simulink model "l8_ver140"  (Simulink 7.9) (continued)
== system l8_ver140 == (continued)
      mask init: same as l8_ver140/adcsnap0
      mask StyleString: popup(bram|dram),popup(1|2|3|4),popup(200|250|266|300|333),edit,popup(8|16|32|64|128),checkbox,checkbox,checkbox,checkbox
      mask CallbackString: snapshot_callback();||||||||
  s5 : Subsystem  mask{mult_latency=6, add_latency=2, bram_length=10}
      mask prompts: mult_latency "Multiplier Latency"; add_latency "Adder Latency"; bram_length "BRAM Length(2^n)"
  s6 : Subsystem  mask{mult_latency=6, add_latency=2, bram_length=10}
      mask prompts: mult_latency "Multiplier Latency"; add_latency "Adder Latency"; bram_length "BRAM Length(2^n)"
  s67snap : Subsystem  Tag=casper:snapshot  AncestorBlock=casper_library_scopes/snapshot  mask(snapshot){storage=bram, dram_dimm=1, dram_clock=200, nsamples=12, data_width=64, offset=off, circap=off, value=off, use_dsp48=on}
      mask prompts: storage "Storage medium"; dram_dimm "DRAM dimm"; dram_clock "DRAM clock rate"; nsamples "Number of Samples (2^?)"; data_width "Data width"; offset "Start delay support"; circap "Circular capture support"; value "Extra value capture support"; use_dsp48 "Use DSP48s to implement counters"
      mask help:
      | Standard snap block, but with the following optional extras;
      |  1) Optionally delay the fabric trigger by a user-specified number of valids.
      |  2) Capture continuously after a trigger until a stop pulse is received. Data is captured into BRAM continuously, with old data being overwritten until a stop command is received. Then stop capturing when memory is filled. This enables you to get some data before and some data after the stop pulse. Use the 'tr_en_cnt' register to determine where you are in your vector. 'Addr' register's MSb now indicates 'busy capturing'.
      |  3) Optionally capture a value on an input port to a register at the same time as the first data sample.
      |  4) Implement counters using DSP48Es to save resources.
      mask init: same as l8_ver140/adcsnap0
      mask StyleString: popup(bram|dram),popup(1|2|3|4),popup(200|250|266|300|333),edit,popup(8|16|32|64|128),checkbox,checkbox,checkbox,checkbox
      mask CallbackString: snapshot_callback();||||||||
  s7 : Subsystem  mask{mult_latency=6, add_latency=2, bram_length=10}
      mask prompts: mult_latency "Multiplier Latency"; add_latency "Adder Latency"; bram_length "BRAM Length(2^n)"
  sg : Subsystem
  ssg : Subsystem
  status : Subsystem  Tag=xps:sw_reg  AncestorBlock=xps_library/software register  mask{numios=1, io_dir=From Processor, io_delay=0, sample_period=1, name1=reg, bitwidth1=32, arith_type1=Unsigned, bin_pt1=0}
      mask prompts: numios "Num I/Os"; io_dir "I/O direction"; io_delay "I/O delay"; sample_period "Sample period"; name1 "Name"; bitwidth1 "Bitwidth"; arith_type1 "Type"; bin_pt1 "Binary pt"
      mask init:
      | swreg_init(gcb);
      mask StyleString: edit,popup(To Processor|From Processor),edit,edit,edit,edit,popup(Unsigned|Signed  (2's comp)|Boolean),edit
      mask CallbackString:
      | t = timer('StartDelay', 0.1); t.TimerFcn = {@swreg_cb, gcb}; start(t);|a = get_param(gcb, 'MaskEnables');
      | if strcmp(get_param(gcb, 'io_dir'), 'To Processor'),
      | a(4) = {'off'};
      | else
      | a(4) = {'on'};
      | end
      | set_param(gcb, 'MaskEnables', a);
      | |||||a = get_param(gcb, 'MaskEnables');
      | if strcmp(get_param(gcb, 'arith_type1'), 'Boolean'),
      | set_param(gcb, 'bitwidth1', '1');
      | set_param(gcb, 'bin_pt1', '0');
      | a(6) = {'off'};
      | a(8) = {'off'};
      | else
      | a(6) = {'on'};
      | a(8) = {'on'};
      | end
      | set_param(gcb, 'MaskEnables', a);
      | |
      mask TabNameString: Setup,Setup,Setup,Setup,IO1,IO1,IO1,IO1
  subband_sampler : Subsystem  mask{subband=1}
      mask prompts: subband "Sub-band number (1 - 8)"
      mask init:
      | if ((subband < 1) || (subband > 8))
      |     err_msg = sprintf('Sub-band number should be in [1, 8]!');
      |     errordlg(err_msg);
      | end
      | 
      | if (mod(subband, 2) == 1)   % odd
      |     offset = 32 * subband;
      | else                        % even
      |     offset = 32 * (subband - 2);
      | end
  trig : Subsystem  Tag=xps:sw_reg  AncestorBlock=xps_library/software register  mask{numios=1, io_dir=From Processor, io_delay=0, sample_period=1, name1=reg, bitwidth1=32, arith_type1=Unsigned, bin_pt1=0}
      mask prompts: numios "Num I/Os"; io_dir "I/O direction"; io_delay "I/O delay"; sample_period "Sample period"; name1 "Name"; bitwidth1 "Bitwidth"; arith_type1 "Type"; bin_pt1 "Binary pt"
      mask init:
      | swreg_init(gcb);
      mask StyleString: edit,popup(To Processor|From Processor),edit,edit,edit,edit,popup(Unsigned|Signed  (2's comp)|Boolean),edit
      mask CallbackString:
      | t = timer('StartDelay', 0.1); t.TimerFcn = {@swreg_cb, gcb}; start(t);|a = get_param(gcb, 'MaskEnables');
      | if strcmp(get_param(gcb, 'io_dir'), 'To Processor'),
      | a(4) = {'off'};
      | else
      | a(4) = {'on'};
      | end
      | set_param(gcb, 'MaskEnables', a);
      | |||||a = get_param(gcb, 'MaskEnables');
      | if strcmp(get_param(gcb, 'arith_type1'), 'Boolean'),
      | set_param(gcb, 'bitwidth1', '1');
      | set_param(gcb, 'bin_pt1', '0');
      | a(6) = {'off'};
      | a(8) = {'off'};
      | else
      | a(6) = {'on'};
      | a(8) = {'on'};
      | end
      | set_param(gcb, 'MaskEnables', a);
      | |
      mask TabNameString: Setup,Setup,Setup,Setup,IO1,IO1,IO1,IO1
  tvg_en : Subsystem  Tag=xps:sw_reg  AncestorBlock=xps_library/software register  mask{numios=1, io_dir=From Processor, io_delay=0, sample_period=1, name1=reg, bitwidth1=32, arith_type1=Unsigned, bin_pt1=0}
      mask prompts: numios "Num I/Os"; io_dir "I/O direction"; io_delay "I/O delay"; sample_period "Sample period"; name1 "Name"; bitwidth1 "Bitwidth"; arith_type1 "Type"; bin_pt1 "Binary pt"
      mask init:
      | swreg_init(gcb);
      mask StyleString: edit,popup(To Processor|From Processor),edit,edit,edit,edit,popup(Unsigned|Signed  (2's comp)|Boolean),edit
      mask CallbackString:
      | t = timer('StartDelay', 0.1); t.TimerFcn = {@swreg_cb, gcb}; start(t);|a = get_param(gcb, 'MaskEnables');
      | if strcmp(get_param(gcb, 'io_dir'), 'To Processor'),
      | a(4) = {'off'};
      | else
      | a(4) = {'on'};
      | end
      | set_param(gcb, 'MaskEnables', a);
      | |||||a = get_param(gcb, 'MaskEnables');
      | if strcmp(get_param(gcb, 'arith_type1'), 'Boolean'),
      | set_param(gcb, 'bitwidth1', '1');
      | set_param(gcb, 'bin_pt1', '0');
      | a(6) = {'off'};
      | a(8) = {'off'};
      | else
      | a(6) = {'on'};
      | a(8) = {'on'};
      | end
      | set_param(gcb, 'MaskEnables', a);
      | |
      mask TabNameString: Setup,Setup,Setup,Setup,IO1,IO1,IO1,IO1
  valid_gen : Subsystem
  xblocks : Subsystem  AncestorBlock=gbt_library/gen_rcs  mask(gen_rcs){lib_name=xblocks_devel, lib_src=git}
      mask prompts: lib_name "Library name"; lib_src "source of revision info"
      mask help:
      | Embeds revision control information for the user design and libraries.
      | On failure to locate specified revision information, falls back to
      | timestamps (current time for libraries).
      | A user specified value is also stored.
      mask init:
      | gen_rcs_init(gcb, ...
      |     'lib_name', lib_name, ...
      |     'lib_src', lib_src);
      settings: AttributesFormatString=|
      | /export/home/ptcs/scratch/models/gjones/xblocks_devel/xblocks_library
      | 8A521D5 : dirty
      mask StyleString: edit,popup(timestamp|git|svn)
  wires:
  Delay54/1 -> [pol1_a0]
  Delay/1 -> converter/1(u8_0)
  Delay2/1 -> converter1/1(u8_0)
  Delay3/1 -> converter2/1(u8_0)
  Delay4/1 -> converter3/1(u8_0)
  Delay5/1 -> converter4/1(u8_0)
  Delay6/1 -> converter5/1(u8_0)
  Delay7/1 -> converter6/1(u8_0)
  Delay8/1 -> converter7/1(u8_0)
  converter/1(s8_7) -> Delay54/1
  converter1/1(s8_7) -> Delay67/1
  converter2/1(s8_7) -> Delay68/1
  converter3/1(s8_7) -> Delay69/1
  converter4/1(s8_7) -> Delay70/1
  converter5/1(s8_7) -> Delay74/1
  converter6/1(s8_7) -> Delay75/1
  converter7/1(s8_7) -> Delay76/1
  Counter/1 -> Slice2/1
  Slice2/1 -> edge_detect1/1
  edge_detect1/1 -> [cic_sync]
  gbe0_overflow/1(sim_out) -> Terminator5/1
  Constant1/1 -> dest_ip/1(sim_reg)
  Constant7/1 -> dest_port/1(sim_reg)
  dest_ip/1(in_reg) -> [dest_ip]
  dest_port/1(in_reg) -> [dest_port]
  [dest_ip] -> gbe0/4
  [dest_port] -> Slice4/1
  Constant2/1 -> status/1(sim_reg)
  status/1(in_reg) -> [status]
  packetizer/3(pkt_eof) -> gbe0/6, [pkt_eof]
  [overflow] -> gbe0_overflow/1(out_reg)
  [spead_rst] -> packetizer/1(reset)
  [status_bits] -> packetizer/2(status)
  Reinterpret8/1 -> [cic_pol1_re], Slice3/1
  Slice3/1 -> [s1_pol1_re]
  Slice5/1 -> [s1_pol1_im]
  Slice6/1 -> [s1_pol2_re]
  Slice7/1 -> [s1_pol2_im]
  reset/1(in_reg) -> [reset]  "reset"
  Slice4/1 -> gbe0/5
  gbe0/6 -> Terminator4/1
  gbe0/7 -> Terminator6/1
  gbe0/8 -> Terminator7/1
  gbe0/9 -> Terminator8/1
  gbe0/10 -> Terminator11/1
  gbe0/11 -> Terminator3/1
  Constant11/1 -> gbe0/7
  gbe0/1 -> Terminator14/1
  gbe0/2 -> Terminator15/1
  gbe0/3 -> Terminator16/1
  gbe0/4 -> Terminator17/1
  gbe0/5 -> negedge_delay/1
  Constant12/1 -> gbe0/8
  gbe0/12 -> Terminator12/1
  [gbe_rst] -> gbe0/1
  negedge_delay/1 -> [overflow]
  valid_gen/1(valid) -> [valid]
  Constant10/1 -> reset/1(sim_reg)
  Constant31/1 -> Register18/1
  sg/2(pps_out) -> Register18/3, [master_rst1]
  Register18/1 -> Inverter2/1, Inverter1/1, [data_enable]  "data_enable"
  arm/1(in_reg) -> Slice8/1
  Pulse Generator/1 -> arm/1(sim_reg)
  Inverter1/1 -> sg/2(pps_en)
  edge_detect2/1 -> Logical3/2
  Slice11/1 -> Logical3/1
  Slice9/1 -> Logical/2
  Logical3/1 -> Logical/3
  [arm] -> Register18/2
  [adc_sync] -> sg/1(pps)
  sg/1(sync_out) -> [sync_out]
  Inverter2/1 -> [spead_rst]  "spead_rst"
  Logical/1 -> [gbe_rst]  "gbe_rst"
  [overflow] -> edge_detect2/1
  [reset] -> Slice9/1, Slice11/1
  Delay21/1 -> Slice10/1
  Slice10/1 -> edge_detect/1
  edge_detect/1 -> [adc_sync]  "adc_sync"
  [spead_rst] -> valid_gen/2(reset)
  Slice8/1 -> [arm]  "arm"
  [arm] -> edge_detect4/1
  [valid] -> Register268/1
  [data_enable] -> Register267/1
  reshaper/1(data_out) -> packetizer/3(data), [reshaper_dout]
  reshaper/2(data_valid) -> packetizer/4(data_valid), [reshaper_valid]
  Reinterpret9/1 -> Slice5/1, [cic_pol1_im]
  Reinterpret10/1 -> [cic_pol2_re], Slice6/1
  Reinterpret11/1 -> Slice7/1, [cic_pol2_im]
  Constant4/1 -> trig/1(sim_reg)
  trig/1(in_reg) -> Slice19/1
  packetizer/1(pkt_data) -> gbe0/2, [pktizer_dout]
  packetizer/2(pkt_valid) -> [pkt_valid], Logical1/2
  [s0_pol1_re] -> Concat1/1
  [s0_pol1_im] -> Concat1/2
  [s0_pol2_re] -> Concat1/3
  [s0_pol2_im] -> Concat1/4
  Concat6/1 -> Concat/1, s67snap/1(din)
  Concat5/1 -> Concat/2, s45snap/1(din)
  Concat2/1 -> Concat/3, s23snap/1(din)
  Concat1/1 -> Concat/4, s01snap/1(din)
  Delay38/1 -> [pol2_a0]
  Delay40/1 -> [pol2_a2]
  Delay41/1 -> [pol2_a4]
  Delay42/1 -> [pol2_a6]
  Delay44/1 -> [pol2_a8]
  Delay45/1 -> [pol2_a10]
  Delay46/1 -> [pol2_a12]
  Delay47/1 -> [pol2_a14]
  Delay25/1 -> converter8/1(u8_0)
  Delay33/1 -> converter9/1(u8_0)
  Delay35/1 -> converter10/1(u8_0)
  Delay36/1 -> converter11/1(u8_0)
  Delay37/1 -> converter12/1(u8_0)
  Delay39/1 -> converter13/1(u8_0)
  Delay43/1 -> converter14/1(u8_0)
  Delay48/1 -> converter15/1(u8_0)
  converter8/1(s8_7) -> Delay38/1
  converter9/1(s8_7) -> Delay40/1
  converter10/1(s8_7) -> Delay41/1
  converter11/1(s8_7) -> Delay42/1
  converter12/1(s8_7) -> Delay44/1
  converter13/1(s8_7) -> Delay45/1
  converter14/1(s8_7) -> Delay46/1
  converter15/1(s8_7) -> Delay47/1
  asiaa_adc5g/2(a1) -> Delay9/1
  asiaa_adc5g/4(a3) -> Delay13/1
  asiaa_adc5g/6(a5) -> Delay15/1
  asiaa_adc5g/8(a7) -> Delay17/1
  asiaa_adc5g/10(a9) -> Delay26/1
  asiaa_adc5g/12(a11) -> Delay28/1
  asiaa_adc5g/14(a13) -> Delay29/1
  asiaa_adc5g/16(a15) -> Delay62/1
  asiaa_adc5g/1(a0) -> Delay/1
  asiaa_adc5g/15(a14) -> Delay8/1
  asiaa_adc5g/13(a12) -> Delay7/1
  asiaa_adc5g/11(a10) -> Delay6/1
  asiaa_adc5g/7(a6) -> Delay4/1
  asiaa_adc5g/5(a4) -> Delay3/1
  asiaa_adc5g/3(a2) -> Delay2/1
  asiaa_adc5g/9(a8) -> Delay5/1
  asiaa_adc5g/17(sync_out) -> Delay21/1
  asiaa_adc5g1/1(a0) -> Delay25/1
  asiaa_adc5g1/2(a1) -> Delay31/1
  asiaa_adc5g1/3(a2) -> Delay33/1
  asiaa_adc5g1/4(a3) -> Delay34/1
  asiaa_adc5g1/5(a4) -> Delay35/1
  asiaa_adc5g1/6(a5) -> Delay50/1
  asiaa_adc5g1/7(a6) -> Delay36/1
  asiaa_adc5g1/8(a7) -> Delay52/1
  asiaa_adc5g1/9(a8) -> Delay37/1
  asiaa_adc5g1/10(a9) -> Delay55/1
  asiaa_adc5g1/11(a10) -> Delay39/1
  asiaa_adc5g1/12(a11) -> Delay57/1
  asiaa_adc5g1/13(a12) -> Delay43/1
  asiaa_adc5g1/14(a13) -> Delay59/1
  asiaa_adc5g1/15(a14) -> Delay48/1
  asiaa_adc5g1/16(a15) -> Delay64/1
  Delay11/1 -> [pol1_a1]
  Delay9/1 -> converter16/1(u8_0)
  converter16/1(s8_7) -> Delay11/1
  Delay13/1 -> converter17/1(u8_0)
  converter17/1(s8_7) -> Delay12/1
  Delay15/1 -> converter18/1(u8_0)
  converter18/1(s8_7) -> Delay14/1
  Delay17/1 -> converter19/1(u8_0)
  converter19/1(s8_7) -> Delay16/1
  Delay26/1 -> converter20/1(u8_0)
  converter20/1(s8_7) -> Delay23/1
  Delay28/1 -> converter21/1(u8_0)
  converter21/1(s8_7) -> Delay27/1
  Delay29/1 -> converter22/1(u8_0)
  converter22/1(s8_7) -> Delay30/1
  Delay32/1 -> [pol2_a1]
  Delay31/1 -> converter23/1(u8_0)
  converter23/1(s8_7) -> Delay32/1
  Delay49/1 -> [pol2_a3]
  Delay34/1 -> converter24/1(u8_0)
  converter24/1(s8_7) -> Delay49/1
  Delay51/1 -> [pol2_a5]
  Delay50/1 -> converter25/1(u8_0)
  converter25/1(s8_7) -> Delay51/1
  Delay53/1 -> [pol2_a7]
  Delay52/1 -> converter26/1(u8_0)
  converter26/1(s8_7) -> Delay53/1
  Delay56/1 -> [pol2_a9]
  Delay55/1 -> converter27/1(u8_0)
  converter27/1(s8_7) -> Delay56/1
  Delay58/1 -> [pol2_a11]
  Delay57/1 -> converter28/1(u8_0)
  converter28/1(s8_7) -> Delay58/1
  Delay60/1 -> [pol2_a13]
  Delay59/1 -> converter29/1(u8_0)
  converter29/1(s8_7) -> Delay60/1
  Delay62/1 -> converter30/1(u8_0)
  converter30/1(s8_7) -> Delay61/1
  Delay63/1 -> [pol2_a15]
  Delay64/1 -> converter31/1(u8_0)
  converter31/1(s8_7) -> Delay63/1
  s1/2(pol1_im) -> Reinterpret9/1
  s1/1(pol1_re) -> Reinterpret8/1
  s1/3(pol2_re) -> Reinterpret10/1
  s1/4(pol2_im) -> Reinterpret11/1
  [cic_sync] -> Register34/1
  [pol1_a0] -> Register1/1
  Constant23/1 -> adcsnap0/2(we)
  bus_create1/1(bus_out) -> adcsnap0/1(din)
  Constant24/1 -> adcsnap1/2(we)
  bus_create2/1(bus_out) -> adcsnap1/1(din)
  [pkt_valid] -> packetizer_snap/2(we)
  [pktizer_dout] -> packetizer_snap/1(din)
  Slice19/1 -> edge_detect3/1
  edge_detect3/1 -> [trig]
  [trig] -> adcsnap0/3(trig)
  [trig] -> adcsnap1/3(trig)
  [trig] -> packetizer_snap/3(trig)
  [s1_pol1_re] -> Concat1/5
  [s1_pol1_im] -> Concat1/6
  [s1_pol2_re] -> Concat1/7
  [s1_pol2_im] -> Concat1/8
  [s2_pol1_re] -> Concat2/1
  [s2_pol1_im] -> Concat2/2
  [s2_pol2_re] -> Concat2/3
  [s2_pol2_im] -> Concat2/4
  [s3_pol1_re] -> Concat2/5
  [s3_pol1_im] -> Concat2/6
  [s3_pol2_re] -> Concat2/7
  [s3_pol2_im] -> Concat2/8
  [s6_pol1_re] -> Concat6/1
  [s6_pol1_im] -> Concat6/2
  [s6_pol2_re] -> Concat6/3
  [s6_pol2_im] -> Concat6/4
  [s7_pol1_re] -> Concat6/5
  [s7_pol1_im] -> Concat6/6
  [s7_pol2_re] -> Concat6/7
  [s7_pol2_im] -> Concat6/8
  [s4_pol1_re] -> Concat5/1
  [s4_pol1_im] -> Concat5/2
  [s4_pol2_re] -> Concat5/3
  [s4_pol2_im] -> Concat5/4
  [s5_pol1_re] -> Concat5/5
  [s5_pol1_im] -> Concat5/6
  [s5_pol2_re] -> Concat5/7
  [s5_pol2_im] -> Concat5/8
  Constant3/1 -> mode_sel/1(sim_reg)
  mode_sel/1(in_reg) -> Slice40/1
  Slice40/1 -> [mode_sel]
  [mode_sel] -> valid_gen/1(mode_sel)
  Mux/1 -> Register266/1
  [mode_sel] -> Mux/1
  subband_sampler/1(data_out) -> Mux/3
  [mode_sel] -> packetizer/5(mode_sel)
  Delay67/1 -> [pol1_a2]
  Delay12/1 -> [pol1_a3]
  Delay68/1 -> [pol1_a4]
  Delay14/1 -> [pol1_a5]
  Delay69/1 -> [pol1_a6]
  Delay16/1 -> [pol1_a7]
  Delay70/1 -> [pol1_a8]
  Delay23/1 -> [pol1_a9]
  Delay74/1 -> [pol1_a10]
  Delay27/1 -> [pol1_a11]
  Delay75/1 -> [pol1_a12]
  Delay30/1 -> [pol1_a13]
  Delay76/1 -> [pol1_a14]
  Delay61/1 -> [pol1_a15]
  [pol1_a1] -> Register2/1
  [pol1_a2] -> Register3/1
  [pol1_a3] -> Register4/1
  [pol1_a4] -> Register5/1
  [pol1_a5] -> Register6/1
  [pol1_a6] -> Register7/1
  [pol1_a7] -> Register8/1
  [pol1_a8] -> Register9/1
  [pol1_a9] -> Register10/1
  [pol1_a10] -> Register11/1
  [pol1_a11] -> Register12/1
  [pol1_a12] -> Register13/1
  [pol1_a13] -> Register14/1
  [pol1_a14] -> Register15/1
  [pol1_a15] -> Register16/1
  [pol2_a0] -> Register17/1
  [pol2_a1] -> Register26/1
  [pol2_a2] -> Register27/1
  [pol2_a3] -> Register28/1
  [pol2_a4] -> Register29/1
  [pol2_a5] -> Register30/1
  [pol2_a6] -> Register31/1
  [pol2_a7] -> Register32/1
  [pol2_a8] -> Register33/1
  [pol2_a9] -> Register19/1
  [pol2_a10] -> Register20/1
  [pol2_a11] -> Register21/1
  [pol2_a12] -> Register22/1
  [pol2_a13] -> Register23/1
  [pol2_a14] -> Register24/1
  [pol2_a15] -> Register25/1
  [pol1_a0] -> bus_create1/1(in1)
  [pol1_a1] -> bus_create1/2(in2)
  [pol1_a2] -> bus_create1/3(in3)
  [pol1_a3] -> bus_create1/4(in4)
  [pol1_a4] -> bus_create1/5(in5)
  [pol1_a5] -> bus_create1/6(in6)
  [pol1_a6] -> bus_create1/7(in7)
  [pol1_a7] -> bus_create1/8(in8)
  [pol1_a8] -> bus_create1/9(in9)
  [pol1_a9] -> bus_create1/10(in10)
  [pol1_a10] -> bus_create1/11(in11)
  [pol1_a11] -> bus_create1/12(in12)
  [pol1_a12] -> bus_create1/13(in13)
  [pol1_a13] -> bus_create1/14(in14)
  [pol1_a14] -> bus_create1/15(in15)
  [pol1_a15] -> bus_create1/16(in16)
  [pol2_a0] -> bus_create2/1(in1)
  [pol2_a1] -> bus_create2/2(in2)
  [pol2_a2] -> bus_create2/3(in3)
  [pol2_a3] -> bus_create2/4(in4)
  [pol2_a4] -> bus_create2/5(in5)
  [pol2_a5] -> bus_create2/6(in6)
  [pol2_a6] -> bus_create2/7(in7)
  [pol2_a7] -> bus_create2/8(in8)
  [pol2_a8] -> bus_create2/9(in9)
  [pol2_a9] -> bus_create2/10(in10)
  [pol2_a10] -> bus_create2/11(in11)
  [pol2_a11] -> bus_create2/12(in12)
  [pol2_a12] -> bus_create2/13(in13)
  [pol2_a13] -> bus_create2/14(in14)
  [pol2_a14] -> bus_create2/15(in15)
  [pol2_a15] -> bus_create2/16(in16)
  ssg/1(statusbits) -> [status_bits]
  [master_rst1] -> Register/1
  Register/1 -> ssg/1(reset)
  [spec_tick] -> ssg/2(spec_tick)
  [master_rst1] -> Counter1/1
  Counter1/1 -> Slice41/1
  Slice41/1 -> edge_detect5/1(in)
  edge_detect5/1(out) -> [spec_tick]
  Reinterpret3/1 -> Slice1/1
  Slice1/1 -> [s0_pol1_re]
  Slice12/1 -> [s0_pol1_im]
  Slice13/1 -> [s0_pol2_re]
  Slice14/1 -> [s0_pol2_im]
  Reinterpret4/1 -> Slice12/1
  Reinterpret1/1 -> Slice13/1
  Reinterpret2/1 -> Slice14/1
  s0/2(pol1_im) -> Reinterpret4/1
  s0/1(pol1_re) -> Reinterpret3/1
  s0/3(pol2_re) -> Reinterpret1/1
  s0/4(pol2_im) -> Reinterpret2/1
  Reinterpret7/1 -> Slice15/1
  Slice15/1 -> [s2_pol1_re]
  Slice16/1 -> [s2_pol1_im]
  Slice17/1 -> [s2_pol2_re]
  Slice18/1 -> [s2_pol2_im]
  Reinterpret12/1 -> Slice16/1
  Reinterpret5/1 -> Slice17/1
  Reinterpret6/1 -> Slice18/1
  s2/2(pol1_im) -> Reinterpret12/1
  s2/1(pol1_re) -> Reinterpret7/1
  s2/3(pol2_re) -> Reinterpret5/1
  s2/4(pol2_im) -> Reinterpret6/1
  Reinterpret16/1 -> Slice20/1
  Slice20/1 -> [s3_pol1_re]
  Slice21/1 -> [s3_pol1_im]
  Slice22/1 -> [s3_pol2_re]
  Slice23/1 -> [s3_pol2_im]
  Reinterpret13/1 -> Slice21/1
  Reinterpret14/1 -> Slice22/1
  Reinterpret15/1 -> Slice23/1
  s3/2(pol1_im) -> Reinterpret13/1
  s3/1(pol1_re) -> Reinterpret16/1
  s3/3(pol2_re) -> Reinterpret14/1
  s3/4(pol2_im) -> Reinterpret15/1
  Reinterpret20/1 -> Slice24/1
  Slice24/1 -> [s4_pol1_re]
  Slice25/1 -> [s4_pol1_im]
  Slice26/1 -> [s4_pol2_re]
  Slice27/1 -> [s4_pol2_im]
  Reinterpret17/1 -> Slice25/1
  Reinterpret18/1 -> Slice26/1
  Reinterpret19/1 -> Slice27/1
  s4/2(pol1_im) -> Reinterpret17/1
  s4/1(pol1_re) -> Reinterpret20/1
  s4/3(pol2_re) -> Reinterpret18/1
  s4/4(pol2_im) -> Reinterpret19/1
  Reinterpret24/1 -> Slice28/1
  Slice28/1 -> [s5_pol1_re]
  Slice29/1 -> [s5_pol1_im]
  Slice30/1 -> [s5_pol2_re]
  Slice31/1 -> [s5_pol2_im]
  Reinterpret21/1 -> Slice29/1
  Reinterpret22/1 -> Slice30/1
  Reinterpret23/1 -> Slice31/1
  s5/2(pol1_im) -> Reinterpret21/1
  s5/1(pol1_re) -> Reinterpret24/1
  s5/3(pol2_re) -> Reinterpret22/1
  s5/4(pol2_im) -> Reinterpret23/1
  Reinterpret28/1 -> Slice32/1
  Slice32/1 -> [s6_pol1_re]
  Slice33/1 -> [s6_pol1_im]
  Slice34/1 -> [s6_pol2_re]
  Slice35/1 -> [s6_pol2_im]
  Reinterpret25/1 -> Slice33/1
  Reinterpret26/1 -> Slice34/1
  Reinterpret27/1 -> Slice35/1
  s6/2(pol1_im) -> Reinterpret25/1
  s6/1(pol1_re) -> Reinterpret28/1
  s6/3(pol2_re) -> Reinterpret26/1
  s6/4(pol2_im) -> Reinterpret27/1
  Reinterpret32/1 -> Slice36/1
  Slice36/1 -> [s7_pol1_re]
  Slice37/1 -> [s7_pol1_im]
  Slice38/1 -> [s7_pol2_re]
  Slice39/1 -> [s7_pol2_im]
  Reinterpret29/1 -> Slice37/1
  Reinterpret30/1 -> Slice38/1
  Reinterpret31/1 -> Slice39/1
  s7/2(pol1_im) -> Reinterpret29/1
  s7/1(pol1_re) -> Reinterpret32/1
  s7/3(pol2_re) -> Reinterpret30/1
  s7/4(pol2_im) -> Reinterpret31/1
  Register1/1 -> s1/1(pol1_in0)
  Register2/1 -> s1/2(pol1_in1)
  Register3/1 -> s1/3(pol1_in2)
  Register4/1 -> s1/4(pol1_in3)
  Register5/1 -> s1/5(pol1_in4)
  Register6/1 -> s1/6(pol1_in5)
  Register7/1 -> s1/7(pol1_in6)
  Register8/1 -> s1/8(pol1_in7)
  Register9/1 -> s1/9(pol1_in8)
  Register10/1 -> s1/10(pol1_in9)
  Register11/1 -> s1/11(pol1_in10)
  Register12/1 -> s1/12(pol1_in11)
  Register13/1 -> s1/13(pol1_in12)
  Register14/1 -> s1/14(pol1_in13)
  Register15/1 -> s1/15(pol1_in14)
  Register16/1 -> s1/16(pol1_in15)
  Register17/1 -> s1/17(pol2_in0)
  Register26/1 -> s1/18(pol2_in1)
  Register27/1 -> s1/19(pol2_in2)
  Register28/1 -> s1/20(pol2_in3)
  Register29/1 -> s1/21(pol2_in4)
  Register30/1 -> s1/22(pol2_in5)
  Register31/1 -> s1/23(pol2_in6)
  Register32/1 -> s1/24(pol2_in7)
  Register33/1 -> s1/25(pol2_in8)
  Register19/1 -> s1/26(pol2_in9)
  Register20/1 -> s1/27(pol2_in10)
  Register21/1 -> s1/28(pol2_in11)
  Register22/1 -> s1/29(pol2_in12)
  Register23/1 -> s1/30(pol2_in13)
  Register24/1 -> s1/31(pol2_in14)
  Register25/1 -> s1/32(pol2_in15)
  Register34/1 -> s1/33(adc_CIC_sync)
  edge_detect4/1 -> Delay329/1
  Delay329/1 -> Logical/1
  Logical1/1 -> gbe0/3
  [data_enable] -> Logical1/1
  [valid] -> s01snap/2(we)
  [cic_sync] -> Register61/1
  [pol1_a0] -> Register35/1
  [pol1_a1] -> Register45/1
  [pol1_a2] -> Register56/1
  [pol1_a3] -> Register62/1
  [pol1_a4] -> Register63/1
  [pol1_a5] -> Register64/1
  [pol1_a6] -> Register65/1
  [pol1_a7] -> Register66/1
  [pol1_a8] -> Register67/1
  [pol1_a9] -> Register36/1
  [pol1_a10] -> Register37/1
  [pol1_a11] -> Register38/1
  [pol1_a12] -> Register39/1
  [pol1_a13] -> Register40/1
  [pol1_a14] -> Register41/1
  [pol1_a15] -> Register42/1
  [pol2_a0] -> Register43/1
  [pol2_a1] -> Register52/1
  [pol2_a2] -> Register53/1
  [pol2_a3] -> Register54/1
  [pol2_a4] -> Register55/1
  [pol2_a5] -> Register57/1
  [pol2_a6] -> Register58/1
  [pol2_a7] -> Register59/1
  [pol2_a8] -> Register60/1
  [pol2_a9] -> Register44/1
  [pol2_a10] -> Register46/1
  [pol2_a11] -> Register47/1
  [pol2_a12] -> Register48/1
  [pol2_a13] -> Register49/1
  [pol2_a14] -> Register50/1
  [pol2_a15] -> Register51/1
  Register35/1 -> s0/1(pol1_in0)
  Register45/1 -> s0/2(pol1_in1)
  Register56/1 -> s0/3(pol1_in2)
  Register62/1 -> s0/4(pol1_in3)
  Register63/1 -> s0/5(pol1_in4)
  Register64/1 -> s0/6(pol1_in5)
  Register65/1 -> s0/7(pol1_in6)
  Register66/1 -> s0/8(pol1_in7)
  Register67/1 -> s0/9(pol1_in8)
  Register36/1 -> s0/10(pol1_in9)
  Register37/1 -> s0/11(pol1_in10)
  Register38/1 -> s0/12(pol1_in11)
  Register39/1 -> s0/13(pol1_in12)
  Register40/1 -> s0/14(pol1_in13)
  Register41/1 -> s0/15(pol1_in14)
  Register42/1 -> s0/16(pol1_in15)
  Register43/1 -> s0/17(pol2_in0)
  Register52/1 -> s0/18(pol2_in1)
  Register53/1 -> s0/19(pol2_in2)
  Register54/1 -> s0/20(pol2_in3)
  Register55/1 -> s0/21(pol2_in4)
  Register57/1 -> s0/22(pol2_in5)
  Register58/1 -> s0/23(pol2_in6)
  Register59/1 -> s0/24(pol2_in7)
  Register60/1 -> s0/25(pol2_in8)
  Register44/1 -> s0/26(pol2_in9)
  Register46/1 -> s0/27(pol2_in10)
  Register47/1 -> s0/28(pol2_in11)
  Register48/1 -> s0/29(pol2_in12)
  Register49/1 -> s0/30(pol2_in13)
  Register50/1 -> s0/31(pol2_in14)
  Register51/1 -> s0/32(pol2_in15)
  Register61/1 -> s0/33(adc_CIC_sync)
  [cic_sync] -> Register94/1
  [pol1_a0] -> Register68/1
  [pol1_a1] -> Register78/1
  [pol1_a2] -> Register89/1
  [pol1_a3] -> Register95/1
  [pol1_a4] -> Register96/1
  [pol1_a5] -> Register97/1
  [pol1_a6] -> Register98/1
  [pol1_a7] -> Register99/1
  [pol1_a8] -> Register100/1
  [pol1_a9] -> Register69/1
  [pol1_a10] -> Register70/1
  [pol1_a11] -> Register71/1
  [pol1_a12] -> Register72/1
  [pol1_a13] -> Register73/1
  [pol1_a14] -> Register74/1
  [pol1_a15] -> Register75/1
  [pol2_a0] -> Register76/1
  [pol2_a1] -> Register85/1
  [pol2_a2] -> Register86/1
  [pol2_a3] -> Register87/1
  [pol2_a4] -> Register88/1
  [pol2_a5] -> Register90/1
  [pol2_a6] -> Register91/1
  [pol2_a7] -> Register92/1
  [pol2_a8] -> Register93/1
  [pol2_a9] -> Register77/1
  [pol2_a10] -> Register79/1
  [pol2_a11] -> Register80/1
  [pol2_a12] -> Register81/1
  [pol2_a13] -> Register82/1
  [pol2_a14] -> Register83/1
  [pol2_a15] -> Register84/1
  Register68/1 -> s3/1(pol1_in0)
  Register78/1 -> s3/2(pol1_in1)
  Register89/1 -> s3/3(pol1_in2)
  Register95/1 -> s3/4(pol1_in3)
  Register96/1 -> s3/5(pol1_in4)
  Register97/1 -> s3/6(pol1_in5)
  Register98/1 -> s3/7(pol1_in6)
  Register99/1 -> s3/8(pol1_in7)
  Register100/1 -> s3/9(pol1_in8)
  Register69/1 -> s3/10(pol1_in9)
  Register70/1 -> s3/11(pol1_in10)
  Register71/1 -> s3/12(pol1_in11)
  Register72/1 -> s3/13(pol1_in12)
  Register73/1 -> s3/14(pol1_in13)
  Register74/1 -> s3/15(pol1_in14)
  Register75/1 -> s3/16(pol1_in15)
  Register76/1 -> s3/17(pol2_in0)
  Register85/1 -> s3/18(pol2_in1)
  Register86/1 -> s3/19(pol2_in2)
  Register87/1 -> s3/20(pol2_in3)
  Register88/1 -> s3/21(pol2_in4)
  Register90/1 -> s3/22(pol2_in5)
  Register91/1 -> s3/23(pol2_in6)
  Register92/1 -> s3/24(pol2_in7)
  Register93/1 -> s3/25(pol2_in8)
  Register77/1 -> s3/26(pol2_in9)
  Register79/1 -> s3/27(pol2_in10)
  Register80/1 -> s3/28(pol2_in11)
  Register81/1 -> s3/29(pol2_in12)
  Register82/1 -> s3/30(pol2_in13)
  Register83/1 -> s3/31(pol2_in14)
  Register84/1 -> s3/32(pol2_in15)
  Register94/1 -> s3/33(adc_CIC_sync)
  [cic_sync] -> Register128/1
  [pol1_a0] -> Register102/1
  [pol1_a1] -> Register112/1
  [pol1_a2] -> Register123/1
  [pol1_a3] -> Register129/1
  [pol1_a4] -> Register130/1
  [pol1_a5] -> Register131/1
  [pol1_a6] -> Register132/1
  [pol1_a7] -> Register133/1
  [pol1_a8] -> Register101/1
  [pol1_a9] -> Register103/1
  [pol1_a10] -> Register104/1
  [pol1_a11] -> Register105/1
  [pol1_a12] -> Register106/1
  [pol1_a13] -> Register107/1
  [pol1_a14] -> Register108/1
  [pol1_a15] -> Register109/1
  [pol2_a0] -> Register110/1
  [pol2_a1] -> Register119/1
  [pol2_a2] -> Register120/1
  [pol2_a3] -> Register121/1
  [pol2_a4] -> Register122/1
  [pol2_a5] -> Register124/1
  [pol2_a6] -> Register125/1
  [pol2_a7] -> Register126/1
  [pol2_a8] -> Register127/1
  [pol2_a9] -> Register111/1
  [pol2_a10] -> Register113/1
  [pol2_a11] -> Register114/1
  [pol2_a12] -> Register115/1
  [pol2_a13] -> Register116/1
  [pol2_a14] -> Register117/1
  [pol2_a15] -> Register118/1
  Register102/1 -> s2/1(pol1_in0)
  Register112/1 -> s2/2(pol1_in1)
  Register123/1 -> s2/3(pol1_in2)
  Register129/1 -> s2/4(pol1_in3)
  Register130/1 -> s2/5(pol1_in4)
  Register131/1 -> s2/6(pol1_in5)
  Register132/1 -> s2/7(pol1_in6)
  Register133/1 -> s2/8(pol1_in7)
  Register101/1 -> s2/9(pol1_in8)
  Register103/1 -> s2/10(pol1_in9)
  Register104/1 -> s2/11(pol1_in10)
  Register105/1 -> s2/12(pol1_in11)
  Register106/1 -> s2/13(pol1_in12)
  Register107/1 -> s2/14(pol1_in13)
  Register108/1 -> s2/15(pol1_in14)
  Register109/1 -> s2/16(pol1_in15)
  Register110/1 -> s2/17(pol2_in0)
  Register119/1 -> s2/18(pol2_in1)
  Register120/1 -> s2/19(pol2_in2)
  Register121/1 -> s2/20(pol2_in3)
  Register122/1 -> s2/21(pol2_in4)
  Register124/1 -> s2/22(pol2_in5)
  Register125/1 -> s2/23(pol2_in6)
  Register126/1 -> s2/24(pol2_in7)
  Register127/1 -> s2/25(pol2_in8)
  Register111/1 -> s2/26(pol2_in9)
  Register113/1 -> s2/27(pol2_in10)
  Register114/1 -> s2/28(pol2_in11)
  Register115/1 -> s2/29(pol2_in12)
  Register116/1 -> s2/30(pol2_in13)
  Register117/1 -> s2/31(pol2_in14)
  Register118/1 -> s2/32(pol2_in15)
  Register128/1 -> s2/33(adc_CIC_sync)
  [cic_sync] -> Register161/1
  [pol1_a0] -> Register135/1
  [pol1_a1] -> Register145/1
  [pol1_a2] -> Register156/1
  [pol1_a3] -> Register162/1
  [pol1_a4] -> Register163/1
  [pol1_a5] -> Register164/1
  [pol1_a6] -> Register165/1
  [pol1_a7] -> Register166/1
  [pol1_a8] -> Register134/1
  [pol1_a9] -> Register136/1
  [pol1_a10] -> Register137/1
  [pol1_a11] -> Register138/1
  [pol1_a12] -> Register139/1
  [pol1_a13] -> Register140/1
  [pol1_a14] -> Register141/1
  [pol1_a15] -> Register142/1
  [pol2_a0] -> Register143/1
  [pol2_a1] -> Register152/1
  [pol2_a2] -> Register153/1
  [pol2_a3] -> Register154/1
  [pol2_a4] -> Register155/1
  [pol2_a5] -> Register157/1
  [pol2_a6] -> Register158/1
  [pol2_a7] -> Register159/1
  [pol2_a8] -> Register160/1
  [pol2_a9] -> Register144/1
  [pol2_a10] -> Register146/1
  [pol2_a11] -> Register147/1
  [pol2_a12] -> Register148/1
  [pol2_a13] -> Register149/1
  [pol2_a14] -> Register150/1
  [pol2_a15] -> Register151/1
  Register135/1 -> s4/1(pol1_in0)
  Register145/1 -> s4/2(pol1_in1)
  Register156/1 -> s4/3(pol1_in2)
  Register162/1 -> s4/4(pol1_in3)
  Register163/1 -> s4/5(pol1_in4)
  Register164/1 -> s4/6(pol1_in5)
  Register165/1 -> s4/7(pol1_in6)
  Register166/1 -> s4/8(pol1_in7)
  Register134/1 -> s4/9(pol1_in8)
  Register136/1 -> s4/10(pol1_in9)
  Register137/1 -> s4/11(pol1_in10)
  Register138/1 -> s4/12(pol1_in11)
  Register139/1 -> s4/13(pol1_in12)
  Register140/1 -> s4/14(pol1_in13)
  Register141/1 -> s4/15(pol1_in14)
  Register142/1 -> s4/16(pol1_in15)
  Register143/1 -> s4/17(pol2_in0)
  Register152/1 -> s4/18(pol2_in1)
  Register153/1 -> s4/19(pol2_in2)
  Register154/1 -> s4/20(pol2_in3)
  Register155/1 -> s4/21(pol2_in4)
  Register157/1 -> s4/22(pol2_in5)
  Register158/1 -> s4/23(pol2_in6)
  Register159/1 -> s4/24(pol2_in7)
  Register160/1 -> s4/25(pol2_in8)
  Register144/1 -> s4/26(pol2_in9)
  Register146/1 -> s4/27(pol2_in10)
  Register147/1 -> s4/28(pol2_in11)
  Register148/1 -> s4/29(pol2_in12)
  Register149/1 -> s4/30(pol2_in13)
  Register150/1 -> s4/31(pol2_in14)
  Register151/1 -> s4/32(pol2_in15)
  Register161/1 -> s4/33(adc_CIC_sync)
  [cic_sync] -> Register194/1
  [pol1_a0] -> Register168/1
  [pol1_a1] -> Register178/1
  [pol1_a2] -> Register189/1
  [pol1_a3] -> Register195/1
  [pol1_a4] -> Register196/1
  [pol1_a5] -> Register197/1
  [pol1_a6] -> Register198/1
  [pol1_a7] -> Register199/1
  [pol1_a8] -> Register167/1
  [pol1_a9] -> Register169/1
  [pol1_a10] -> Register170/1
  [pol1_a11] -> Register171/1
  [pol1_a12] -> Register172/1
  [pol1_a13] -> Register173/1
  [pol1_a14] -> Register174/1
  [pol1_a15] -> Register175/1
  [pol2_a0] -> Register176/1
  [pol2_a1] -> Register185/1
  [pol2_a2] -> Register186/1
  [pol2_a3] -> Register187/1
  [pol2_a4] -> Register188/1
  [pol2_a5] -> Register190/1
  [pol2_a6] -> Register191/1
  [pol2_a7] -> Register192/1
  [pol2_a8] -> Register193/1
  [pol2_a9] -> Register177/1
  [pol2_a10] -> Register179/1
  [pol2_a11] -> Register180/1
  [pol2_a12] -> Register181/1
  [pol2_a13] -> Register182/1
  [pol2_a14] -> Register183/1
  [pol2_a15] -> Register184/1
  Register168/1 -> s5/1(pol1_in0)
  Register178/1 -> s5/2(pol1_in1)
  Register189/1 -> s5/3(pol1_in2)
  Register195/1 -> s5/4(pol1_in3)
  Register196/1 -> s5/5(pol1_in4)
  Register197/1 -> s5/6(pol1_in5)
  Register198/1 -> s5/7(pol1_in6)
  Register199/1 -> s5/8(pol1_in7)
  Register167/1 -> s5/9(pol1_in8)
  Register169/1 -> s5/10(pol1_in9)
  Register170/1 -> s5/11(pol1_in10)
  Register171/1 -> s5/12(pol1_in11)
  Register172/1 -> s5/13(pol1_in12)
  Register173/1 -> s5/14(pol1_in13)
  Register174/1 -> s5/15(pol1_in14)
  Register175/1 -> s5/16(pol1_in15)
  Register176/1 -> s5/17(pol2_in0)
  Register185/1 -> s5/18(pol2_in1)
  Register186/1 -> s5/19(pol2_in2)
  Register187/1 -> s5/20(pol2_in3)
  Register188/1 -> s5/21(pol2_in4)
  Register190/1 -> s5/22(pol2_in5)
  Register191/1 -> s5/23(pol2_in6)
  Register192/1 -> s5/24(pol2_in7)
  Register193/1 -> s5/25(pol2_in8)
  Register177/1 -> s5/26(pol2_in9)
  Register179/1 -> s5/27(pol2_in10)
  Register180/1 -> s5/28(pol2_in11)
  Register181/1 -> s5/29(pol2_in12)
  Register182/1 -> s5/30(pol2_in13)
  Register183/1 -> s5/31(pol2_in14)
  Register184/1 -> s5/32(pol2_in15)
  Register194/1 -> s5/33(adc_CIC_sync)
  [cic_sync] -> Register227/1
  [pol1_a0] -> Register201/1
  [pol1_a1] -> Register211/1
  [pol1_a2] -> Register222/1
  [pol1_a3] -> Register228/1
  [pol1_a4] -> Register229/1
  [pol1_a5] -> Register230/1
  [pol1_a6] -> Register231/1
  [pol1_a7] -> Register232/1
  [pol1_a8] -> Register200/1
  [pol1_a9] -> Register202/1
  [pol1_a10] -> Register203/1
  [pol1_a11] -> Register204/1
  [pol1_a12] -> Register205/1
  [pol1_a13] -> Register206/1
  [pol1_a14] -> Register207/1
  [pol1_a15] -> Register208/1
  [pol2_a0] -> Register209/1
  [pol2_a1] -> Register218/1
  [pol2_a2] -> Register219/1
  [pol2_a3] -> Register220/1
  [pol2_a4] -> Register221/1
  [pol2_a5] -> Register223/1
  [pol2_a6] -> Register224/1
  [pol2_a7] -> Register225/1
  [pol2_a8] -> Register226/1
  [pol2_a9] -> Register210/1
  [pol2_a10] -> Register212/1
  [pol2_a11] -> Register213/1
  [pol2_a12] -> Register214/1
  [pol2_a13] -> Register215/1
  [pol2_a14] -> Register216/1
  [pol2_a15] -> Register217/1
  Register201/1 -> s6/1(pol1_in0)
  Register211/1 -> s6/2(pol1_in1)
  Register222/1 -> s6/3(pol1_in2)
  Register228/1 -> s6/4(pol1_in3)
  Register229/1 -> s6/5(pol1_in4)
  Register230/1 -> s6/6(pol1_in5)
  Register231/1 -> s6/7(pol1_in6)
  Register232/1 -> s6/8(pol1_in7)
  Register200/1 -> s6/9(pol1_in8)
  Register202/1 -> s6/10(pol1_in9)
  Register203/1 -> s6/11(pol1_in10)
  Register204/1 -> s6/12(pol1_in11)
  Register205/1 -> s6/13(pol1_in12)
  Register206/1 -> s6/14(pol1_in13)
  Register207/1 -> s6/15(pol1_in14)
  Register208/1 -> s6/16(pol1_in15)
  Register209/1 -> s6/17(pol2_in0)
  Register218/1 -> s6/18(pol2_in1)
  Register219/1 -> s6/19(pol2_in2)
  Register220/1 -> s6/20(pol2_in3)
  Register221/1 -> s6/21(pol2_in4)
  Register223/1 -> s6/22(pol2_in5)
  Register224/1 -> s6/23(pol2_in6)
  Register225/1 -> s6/24(pol2_in7)
  Register226/1 -> s6/25(pol2_in8)
  Register210/1 -> s6/26(pol2_in9)
  Register212/1 -> s6/27(pol2_in10)
  Register213/1 -> s6/28(pol2_in11)
  Register214/1 -> s6/29(pol2_in12)
  Register215/1 -> s6/30(pol2_in13)
  Register216/1 -> s6/31(pol2_in14)
  Register217/1 -> s6/32(pol2_in15)
  Register227/1 -> s6/33(adc_CIC_sync)
  [cic_sync] -> Register260/1
  [pol1_a0] -> Register234/1
  [pol1_a1] -> Register244/1
  [pol1_a2] -> Register255/1
  [pol1_a3] -> Register261/1
  [pol1_a4] -> Register262/1
  [pol1_a5] -> Register263/1
  [pol1_a6] -> Register264/1
  [pol1_a7] -> Register265/1
  [pol1_a8] -> Register233/1
  [pol1_a9] -> Register235/1
  [pol1_a10] -> Register236/1
  [pol1_a11] -> Register237/1
  [pol1_a12] -> Register238/1
  [pol1_a13] -> Register239/1
  [pol1_a14] -> Register240/1
  [pol1_a15] -> Register241/1
  [pol2_a0] -> Register242/1
  [pol2_a1] -> Register251/1
  [pol2_a2] -> Register252/1
  [pol2_a3] -> Register253/1
  [pol2_a4] -> Register254/1
  [pol2_a5] -> Register256/1
  [pol2_a6] -> Register257/1
  [pol2_a7] -> Register258/1
  [pol2_a8] -> Register259/1
  [pol2_a9] -> Register243/1
  [pol2_a10] -> Register245/1
  [pol2_a11] -> Register246/1
  [pol2_a12] -> Register247/1
  [pol2_a13] -> Register248/1
  [pol2_a14] -> Register249/1
  [pol2_a15] -> Register250/1
  Register234/1 -> s7/1(pol1_in0)
  Register244/1 -> s7/2(pol1_in1)
  Register255/1 -> s7/3(pol1_in2)
  Register261/1 -> s7/4(pol1_in3)
  Register262/1 -> s7/5(pol1_in4)
  Register263/1 -> s7/6(pol1_in5)
  Register264/1 -> s7/7(pol1_in6)
  Register265/1 -> s7/8(pol1_in7)
  Register233/1 -> s7/9(pol1_in8)
  Register235/1 -> s7/10(pol1_in9)
  Register236/1 -> s7/11(pol1_in10)
  Register237/1 -> s7/12(pol1_in11)
  Register238/1 -> s7/13(pol1_in12)
  Register239/1 -> s7/14(pol1_in13)
  Register240/1 -> s7/15(pol1_in14)
  Register241/1 -> s7/16(pol1_in15)
  Register242/1 -> s7/17(pol2_in0)
  Register251/1 -> s7/18(pol2_in1)
  Register252/1 -> s7/19(pol2_in2)
  Register253/1 -> s7/20(pol2_in3)
  Register254/1 -> s7/21(pol2_in4)
  Register256/1 -> s7/22(pol2_in5)
  Register257/1 -> s7/23(pol2_in6)
  Register258/1 -> s7/24(pol2_in7)
  Register259/1 -> s7/25(pol2_in8)
  Register243/1 -> s7/26(pol2_in9)
  Register245/1 -> s7/27(pol2_in10)
  Register246/1 -> s7/28(pol2_in11)
  Register247/1 -> s7/29(pol2_in12)
  Register248/1 -> s7/30(pol2_in13)
  Register249/1 -> s7/31(pol2_in14)
  Register250/1 -> s7/32(pol2_in15)
  Register260/1 -> s7/33(adc_CIC_sync)
  [valid] -> s23snap/2(we)
  [valid] -> s45snap/2(we)
  [valid] -> s67snap/2(we)
  [trig] -> s67snap/3(trig)
  [trig] -> s45snap/3(trig)
  [trig] -> s23snap/3(trig)
  [trig] -> s01snap/3(trig)
  Counter2/1 -> Concat3/16, Concat3/14, Concat3/12, Concat3/10, Concat3/8, Concat3/6, Concat3/4, Concat3/2
  Constant13/1 -> Concat3/13
  Constant15/1 -> Concat3/1
  Constant16/1 -> Concat3/3
  Constant9/1 -> Concat3/5
  Constant14/1 -> Concat3/7
  Constant6/1 -> Concat3/9
  Constant8/1 -> Concat3/11
  Constant5/1 -> Concat3/15
  [spead_rst] -> Counter2/1
  Mux1/1 -> Mux/2, subband_sampler/1(data_in)
  Concat3/1 -> Mux1/3
  Concat/1 -> Mux1/2
  [tvg] -> Mux1/1
  Register266/1 -> reshaper/3(data_in)
  Register267/1 -> reshaper/2(data_enable)
  Register268/1 -> reshaper/1(valid)
  Constant17/1 -> tvg_en/1(sim_reg)
  tvg_en/1(in_reg) -> Register269/1
  Slice42/1 -> [tvg]
  Register269/1 -> Slice42/1
